simulink model "Simulatore_COMAU_UBHAND_IV_manipolazione_interna"  (Simulink 7.6)
settings: Solver=ode45  StartTime=0.0  StopTime=inf  RelTol=1e-3
values from neighbouring files (symbols this model uses):
  a_f = zeros(4,5)    [KinematicData.m]
  alpha_f = zeros(4,5)    [KinematicData.m]
  d_f = zeros(4,5)    [KinematicData.m]
  dc4_1 = 660    [KinematicData.m]
  T6HAND = [1 0 0 0;0 1 0 0; 0 0 1 357.48+95; 0 0 0 1]    [KinematicData.m]
  teta_d = atan(647.07/130)    [KinematicData.m]
  teta_f = zeros(4,5)    [KinematicData.m]
undefined symbols (not defined in the file or in its folder): a_c, a_w, alpha_c, alpha_w, d_c, d_w, Dt_fingers, teta_c
libraries: simulink x281, dspmtrx3 x171, aerolibutil x163, aerolibconvert2 x64, simulink_extras x46, vrlib x26, aerolibtransform2 x7, dspnetwork x1
(part 14 of 15)

== system Simulatore_COMAU_UBHAND_IV_manipolazione_interna/Work Space Control/Grasp Planner/cilindro/punti di contatto cilindro/object rotation matrix/R_01/rot_y ==
  beta : Inport
  Angle Conversion : aerolibconvert2/Angle Conversion  IU=deg  OU=rad
  Constant{1..3,5} (4 alike) : Constant  Value=0
  Constant4 : Constant
  Create 3x3 Matrix : aerolibutil/Create 3x3 Matrix
  Gain : Gain  Gain=-1
  Trigonometric Function : Trigonometry
  Trigonometric Function1 : Trigonometry  Operator=cos
  Trigonometric Function4 : Trigonometry  Operator=cos
  Trigonometric Function5 : Trigonometry
  roty : Outport
  wires:
  beta/1 -> Angle Conversion/1
  Angle Conversion/1 -> Trigonometric Function1/1, Trigonometric Function/1, Trigonometric Function5/1, Trigonometric Function4/1
  Create 3x3 Matrix/1 -> roty/1
  Constant2/1 -> Create 3x3 Matrix/2
  Trigonometric Function5/1 -> Create 3x3 Matrix/3
  Constant5/1 -> Create 3x3 Matrix/4
  Constant4/1 -> Create 3x3 Matrix/5
  Constant3/1 -> Create 3x3 Matrix/6
  Trigonometric Function/1 -> Gain/1
  Trigonometric Function1/1 -> Create 3x3 Matrix/9
  Trigonometric Function4/1 -> Create 3x3 Matrix/1
  Gain/1 -> Create 3x3 Matrix/7
  Constant1/1 -> Create 3x3 Matrix/8

== system Simulatore_COMAU_UBHAND_IV_manipolazione_interna/Work Space Control/Grasp Planner/cilindro/punti di contatto cilindro/object rotation matrix/R_01/rot_z ==
  gamma : Inport
  Angle Conversion : aerolibconvert2/Angle Conversion  IU=deg  OU=rad
  Constant{,1..3} (4 alike) : Constant  Value=0
  Constant4 : Constant
  Create 3x3 Matrix : aerolibutil/Create 3x3 Matrix
  Gain1 : Gain  Gain=-1
  Trigonometric Function3 : Trigonometry  Operator=cos
  Trigonometric Function4 : Trigonometry  Operator=cos
  Trigonometric Function5 : Trigonometry
  Trigonometric Function6 : Trigonometry
  rotz : Outport
  wires:
  gamma/1 -> Angle Conversion/1
  Angle Conversion/1 -> Trigonometric Function3/1, Trigonometric Function6/1, Trigonometric Function5/1, Trigonometric Function4/1
  Create 3x3 Matrix/1 -> rotz/1
  Trigonometric Function4/1 -> Create 3x3 Matrix/1
  Gain1/1 -> Create 3x3 Matrix/2
  Constant/1 -> Create 3x3 Matrix/3
  Trigonometric Function6/1 -> Create 3x3 Matrix/4
  Trigonometric Function3/1 -> Create 3x3 Matrix/5
  Constant2/1 -> Create 3x3 Matrix/6
  Constant1/1 -> Create 3x3 Matrix/7
  Constant3/1 -> Create 3x3 Matrix/8
  Constant4/1 -> Create 3x3 Matrix/9
  Trigonometric Function5/1 -> Gain1/1

== system Simulatore_COMAU_UBHAND_IV_manipolazione_interna/Work Space Control/Grasp Planner/cilindro/punti di contatto cilindro/object rotation matrix/R_56/rot_x ==
  alpha : Inport
  Angle Conversion : aerolibconvert2/Angle Conversion  IU=deg  OU=rad
  Constant{,1..2,5} (4 alike) : Constant  Value=0
  Constant4 : Constant
  Create 3x3 Matrix : aerolibutil/Create 3x3 Matrix
  Gain : Gain  Gain=-1
  Trigonometric Function : Trigonometry
  Trigonometric Function1 : Trigonometry  Operator=cos
  Trigonometric Function2 : Trigonometry
  Trigonometric Function3 : Trigonometry  Operator=cos
  rotx : Outport
  wires:
  alpha/1 -> Angle Conversion/1
  Angle Conversion/1 -> Trigonometric Function3/1, Trigonometric Function2/1, Trigonometric Function/1, Trigonometric Function1/1
  Create 3x3 Matrix/1 -> rotx/1
  Constant4/1 -> Create 3x3 Matrix/1
  Constant2/1 -> Create 3x3 Matrix/2
  Constant/1 -> Create 3x3 Matrix/3
  Constant5/1 -> Create 3x3 Matrix/4
  Trigonometric Function3/1 -> Create 3x3 Matrix/5
  Trigonometric Function2/1 -> Gain/1
  Gain/1 -> Create 3x3 Matrix/6
  Constant1/1 -> Create 3x3 Matrix/7
  Trigonometric Function/1 -> Create 3x3 Matrix/8
  Trigonometric Function1/1 -> Create 3x3 Matrix/9

== system Simulatore_COMAU_UBHAND_IV_manipolazione_interna/Work Space Control/Grasp Planner/cilindro/punti di contatto cilindro/object rotation matrix/R_56/rot_y ==
  beta : Inport
  Angle Conversion : aerolibconvert2/Angle Conversion  IU=deg  OU=rad
  Constant{1..3,5} (4 alike) : Constant  Value=0
  Constant4 : Constant
  Create 3x3 Matrix : aerolibutil/Create 3x3 Matrix
  Gain : Gain  Gain=-1
  Trigonometric Function : Trigonometry
  Trigonometric Function1 : Trigonometry  Operator=cos
  Trigonometric Function4 : Trigonometry  Operator=cos
  Trigonometric Function5 : Trigonometry
  roty : Outport
  wires:
  Constant1/1 -> Create 3x3 Matrix/8
  Gain/1 -> Create 3x3 Matrix/7
  Trigonometric Function4/1 -> Create 3x3 Matrix/1
  Trigonometric Function1/1 -> Create 3x3 Matrix/9
  Trigonometric Function/1 -> Gain/1
  Constant3/1 -> Create 3x3 Matrix/6
  Constant4/1 -> Create 3x3 Matrix/5
  Constant5/1 -> Create 3x3 Matrix/4
  Trigonometric Function5/1 -> Create 3x3 Matrix/3
  Constant2/1 -> Create 3x3 Matrix/2
  Create 3x3 Matrix/1 -> roty/1
  Angle Conversion/1 -> Trigonometric Function4/1, Trigonometric Function5/1, Trigonometric Function/1, Trigonometric Function1/1
  beta/1 -> Angle Conversion/1

== system Simulatore_COMAU_UBHAND_IV_manipolazione_interna/Work Space Control/Grasp Planner/cilindro/punti di contatto cilindro/object rotation matrix/R_56/rot_z ==
  gamma : Inport
  Angle Conversion : aerolibconvert2/Angle Conversion  IU=deg  OU=rad
  Constant{,1..3} (4 alike) : Constant  Value=0
  Constant4 : Constant
  Create 3x3 Matrix : aerolibutil/Create 3x3 Matrix
  Gain1 : Gain  Gain=-1
  Trigonometric Function3 : Trigonometry  Operator=cos
  Trigonometric Function4 : Trigonometry  Operator=cos
  Trigonometric Function5 : Trigonometry
  Trigonometric Function6 : Trigonometry
  rotz : Outport
  wires:
  Trigonometric Function5/1 -> Gain1/1
  Constant4/1 -> Create 3x3 Matrix/9
  Constant3/1 -> Create 3x3 Matrix/8
  Constant1/1 -> Create 3x3 Matrix/7
  Constant2/1 -> Create 3x3 Matrix/6
  Trigonometric Function3/1 -> Create 3x3 Matrix/5
  Trigonometric Function6/1 -> Create 3x3 Matrix/4
  Constant/1 -> Create 3x3 Matrix/3
  Gain1/1 -> Create 3x3 Matrix/2
  Trigonometric Function4/1 -> Create 3x3 Matrix/1
  Create 3x3 Matrix/1 -> rotz/1
  Angle Conversion/1 -> Trigonometric Function4/1, Trigonometric Function5/1, Trigonometric Function6/1, Trigonometric Function3/1
  gamma/1 -> Angle Conversion/1

== system Simulatore_COMAU_UBHAND_IV_manipolazione_interna/Work Space Control/Grasp Planner/cilindro/punti di contatto cilindro/punti di contatto cilindro/Subsystem1/Subsystem1 ==
  R : Inport
  p : Inport  Port=2
  Constant2 : Constant  Value=[0 0 0 1]  VectorParams1D=off
  Matrix Concatenate2 : Concatenate  Mode=Multidimensional array  ConcatenateDimension=2
  Matrix Concatenate3 : Concatenate  Mode=Multidimensional array
  T : Outport
  wires:
  Constant2/1 -> Matrix Concatenate3/2
  Matrix Concatenate2/1 -> Matrix Concatenate3/1
  R/1 -> Matrix Concatenate2/1
  p/1 -> Matrix Concatenate2/2
  Matrix Concatenate3/1 -> T/1

== system Simulatore_COMAU_UBHAND_IV_manipolazione_interna/Work Space Control/Grasp Planner/cilindro/punti di contatto cilindro/punti di contatto cilindro/Subsystem1/T^-1 ==
  T : Inport
  -R'*v : Gain  Gain=-1
  Constant1 : Constant  Value=[0 0 0 1]  VectorParams1D=off
  Matrix Concatenation : Concatenate  Mode=Multidimensional array  ConcatenateDimension=2
  Matrix Concatenation1 : Concatenate  Mode=Multidimensional array
  R : dspmtrx3/Submatrix  RowSpan=Range of rows  RowStartMode=Index  RowStartIndex=1  RowEndMode=Index  RowEndIndex=3  ColSpan=Range of columns  ColStartMode=Index  ColStartIndex=1  ColEndMode=Index  ColEndIndex=3
  R' : PermuteDimensions
  R'*v : Product  Multiplication=Matrix(*)
  v : dspmtrx3/Submatrix  RowSpan=Range of rows  RowStartMode=Index  RowStartIndex=1  RowEndMode=Index  RowEndIndex=3  ColSpan=One column  ColStartMode=Index  ColStartIndex=4  ColEndMode=Index  ColEndIndex=3
  T^-1 : Outport
  wires:
  T/1 -> R/1, v/1
  R/1 -> R'/1
  R'/1 -> R'*v/1, Matrix Concatenation/1
  v/1 -> R'*v/2
  R'*v/1 -> -R'*v/1
  Constant1/1 -> Matrix Concatenation1/2
  Matrix Concatenation/1 -> Matrix Concatenation1/1
  -R'*v/1 -> Matrix Concatenation/2
  Matrix Concatenation1/1 -> T^-1/1

== system Simulatore_COMAU_UBHAND_IV_manipolazione_interna/Work Space Control/Grasp Planner/cilindro/punti di contatto cilindro/punti di contatto cilindro/Subsystem2/Subsystem1 ==
  R : Inport
  p : Inport  Port=2
  Constant2 : Constant  Value=[0 0 0 1]  VectorParams1D=off
  Matrix Concatenate2 : Concatenate  Mode=Multidimensional array  ConcatenateDimension=2
  Matrix Concatenate3 : Concatenate  Mode=Multidimensional array
  T : Outport
  wires:
  Constant2/1 -> Matrix Concatenate3/2
  Matrix Concatenate2/1 -> Matrix Concatenate3/1
  R/1 -> Matrix Concatenate2/1
  p/1 -> Matrix Concatenate2/2
  Matrix Concatenate3/1 -> T/1

== system Simulatore_COMAU_UBHAND_IV_manipolazione_interna/Work Space Control/Grasp Planner/cilindro/punti di contatto cilindro/punti di contatto cilindro/Subsystem2/T^-1 ==
  T : Inport
  -R'*v : Gain  Gain=-1
  Constant1 : Constant  Value=[0 0 0 1]  VectorParams1D=off
  Matrix Concatenation : Concatenate  Mode=Multidimensional array  ConcatenateDimension=2
  Matrix Concatenation1 : Concatenate  Mode=Multidimensional array
  R : dspmtrx3/Submatrix  RowSpan=Range of rows  RowStartMode=Index  RowStartIndex=1  RowEndMode=Index  RowEndIndex=3  ColSpan=Range of columns  ColStartMode=Index  ColStartIndex=1  ColEndMode=Index  ColEndIndex=3
  R' : PermuteDimensions
  R'*v : Product  Multiplication=Matrix(*)
  v : dspmtrx3/Submatrix  RowSpan=Range of rows  RowStartMode=Index  RowStartIndex=1  RowEndMode=Index  RowEndIndex=3  ColSpan=One column  ColStartMode=Index  ColStartIndex=4  ColEndMode=Index  ColEndIndex=3
  T^-1 : Outport
  wires:
  T/1 -> R/1, v/1
  R/1 -> R'/1
  R'/1 -> R'*v/1, Matrix Concatenation/1
  v/1 -> R'*v/2
  R'*v/1 -> -R'*v/1
  Constant1/1 -> Matrix Concatenation1/2
  Matrix Concatenation/1 -> Matrix Concatenation1/1
  -R'*v/1 -> Matrix Concatenation/2
  Matrix Concatenation1/1 -> T^-1/1

== system Simulatore_COMAU_UBHAND_IV_manipolazione_interna/Work Space Control/Grasp Planner/cilindro/punti di contatto cilindro/punti di contatto cilindro/Subsystem3/Subsystem1 ==
  R : Inport
  p : Inport  Port=2
  Constant2 : Constant  Value=[0 0 0 1]  VectorParams1D=off
  Matrix Concatenate2 : Concatenate  Mode=Multidimensional array  ConcatenateDimension=2
  Matrix Concatenate3 : Concatenate  Mode=Multidimensional array
  T : Outport
  wires:
  Matrix Concatenate3/1 -> T/1
  p/1 -> Matrix Concatenate2/2
  R/1 -> Matrix Concatenate2/1
  Matrix Concatenate2/1 -> Matrix Concatenate3/1
  Constant2/1 -> Matrix Concatenate3/2

== system Simulatore_COMAU_UBHAND_IV_manipolazione_interna/Work Space Control/Grasp Planner/cilindro/punti di contatto cilindro/punti di contatto cilindro/Subsystem3/T^-1 ==
  T : Inport
  -R'*v : Gain  Gain=-1
  Constant1 : Constant  Value=[0 0 0 1]  VectorParams1D=off
  Matrix Concatenation : Concatenate  Mode=Multidimensional array  ConcatenateDimension=2
  Matrix Concatenation1 : Concatenate  Mode=Multidimensional array
  R : dspmtrx3/Submatrix  RowSpan=Range of rows  RowStartMode=Index  RowStartIndex=1  RowEndMode=Index  RowEndIndex=3  ColSpan=Range of columns  ColStartMode=Index  ColStartIndex=1  ColEndMode=Index  ColEndIndex=3
  R' : PermuteDimensions
  R'*v : Product  Multiplication=Matrix(*)
  v : dspmtrx3/Submatrix  RowSpan=Range of rows  RowStartMode=Index  RowStartIndex=1  RowEndMode=Index  RowEndIndex=3  ColSpan=One column  ColStartMode=Index  ColStartIndex=4  ColEndMode=Index  ColEndIndex=3
  T^-1 : Outport
  wires:
  Matrix Concatenation1/1 -> T^-1/1
  -R'*v/1 -> Matrix Concatenation/2
  Matrix Concatenation/1 -> Matrix Concatenation1/1
  Constant1/1 -> Matrix Concatenation1/2
  R'*v/1 -> -R'*v/1
  v/1 -> R'*v/2
  R'/1 -> Matrix Concatenation/1, R'*v/1
  R/1 -> R'/1
  T/1 -> v/1, R/1

== system Simulatore_COMAU_UBHAND_IV_manipolazione_interna/Work Space Control/Grasp Planner/cilindro/punti di contatto cilindro/punti di contatto cilindro/Subsystem4/Subsystem1 ==
  R : Inport
  p : Inport  Port=2
  Constant2 : Constant  Value=[0 0 0 1]  VectorParams1D=off
  Matrix Concatenate2 : Concatenate  Mode=Multidimensional array  ConcatenateDimension=2
  Matrix Concatenate3 : Concatenate  Mode=Multidimensional array
  T : Outport
  wires:
  Constant2/1 -> Matrix Concatenate3/2
  Matrix Concatenate2/1 -> Matrix Concatenate3/1
  R/1 -> Matrix Concatenate2/1
  p/1 -> Matrix Concatenate2/2
  Matrix Concatenate3/1 -> T/1

== system Simulatore_COMAU_UBHAND_IV_manipolazione_interna/Work Space Control/Grasp Planner/cilindro/punti di contatto cilindro/punti di contatto cilindro/Subsystem4/T^-1 ==
  T : Inport
  -R'*v : Gain  Gain=-1
  Constant1 : Constant  Value=[0 0 0 1]  VectorParams1D=off
  Matrix Concatenation : Concatenate  Mode=Multidimensional array  ConcatenateDimension=2
  Matrix Concatenation1 : Concatenate  Mode=Multidimensional array
  R : dspmtrx3/Submatrix  RowSpan=Range of rows  RowStartMode=Index  RowStartIndex=1  RowEndMode=Index  RowEndIndex=3  ColSpan=Range of columns  ColStartMode=Index  ColStartIndex=1  ColEndMode=Index  ColEndIndex=3
  R' : PermuteDimensions
  R'*v : Product  Multiplication=Matrix(*)
  v : dspmtrx3/Submatrix  RowSpan=Range of rows  RowStartMode=Index  RowStartIndex=1  RowEndMode=Index  RowEndIndex=3  ColSpan=One column  ColStartMode=Index  ColStartIndex=4  ColEndMode=Index  ColEndIndex=3
  T^-1 : Outport
  wires:
  T/1 -> R/1, v/1
  R/1 -> R'/1
  R'/1 -> R'*v/1, Matrix Concatenation/1
  v/1 -> R'*v/2
  R'*v/1 -> -R'*v/1
  Constant1/1 -> Matrix Concatenation1/2
  Matrix Concatenation/1 -> Matrix Concatenation1/1
  -R'*v/1 -> Matrix Concatenation/2
  Matrix Concatenation1/1 -> T^-1/1

== system Simulatore_COMAU_UBHAND_IV_manipolazione_interna/Work Space Control/Grasp Planner/cilindro/punti di contatto cilindro/punti di contatto cilindro/Subsystem5/Subsystem1 ==
  R : Inport
  p : Inport  Port=2
  Constant2 : Constant  Value=[0 0 0 1]  VectorParams1D=off
  Matrix Concatenate2 : Concatenate  Mode=Multidimensional array  ConcatenateDimension=2
  Matrix Concatenate3 : Concatenate  Mode=Multidimensional array
  T : Outport
  wires:
  Matrix Concatenate3/1 -> T/1
  p/1 -> Matrix Concatenate2/2
  R/1 -> Matrix Concatenate2/1
  Matrix Concatenate2/1 -> Matrix Concatenate3/1
  Constant2/1 -> Matrix Concatenate3/2

== system Simulatore_COMAU_UBHAND_IV_manipolazione_interna/Work Space Control/Grasp Planner/cilindro/punti di contatto cilindro/punti di contatto cilindro/Subsystem5/T^-1 ==
  T : Inport
  -R'*v : Gain  Gain=-1
  Constant1 : Constant  Value=[0 0 0 1]  VectorParams1D=off
  Matrix Concatenation : Concatenate  Mode=Multidimensional array  ConcatenateDimension=2
  Matrix Concatenation1 : Concatenate  Mode=Multidimensional array
  R : dspmtrx3/Submatrix  RowSpan=Range of rows  RowStartMode=Index  RowStartIndex=1  RowEndMode=Index  RowEndIndex=3  ColSpan=Range of columns  ColStartMode=Index  ColStartIndex=1  ColEndMode=Index  ColEndIndex=3
  R' : PermuteDimensions
  R'*v : Product  Multiplication=Matrix(*)
  v : dspmtrx3/Submatrix  RowSpan=Range of rows  RowStartMode=Index  RowStartIndex=1  RowEndMode=Index  RowEndIndex=3  ColSpan=One column  ColStartMode=Index  ColStartIndex=4  ColEndMode=Index  ColEndIndex=3
  T^-1 : Outport
  wires:
  Matrix Concatenation1/1 -> T^-1/1
  -R'*v/1 -> Matrix Concatenation/2
  Matrix Concatenation/1 -> Matrix Concatenation1/1
  Constant1/1 -> Matrix Concatenation1/2
  R'*v/1 -> -R'*v/1
  v/1 -> R'*v/2
  R'/1 -> Matrix Concatenation/1, R'*v/1
  R/1 -> R'/1
  T/1 -> v/1, R/1

== system Simulatore_COMAU_UBHAND_IV_manipolazione_interna/Work Space Control/Grasp Planner/sfera/punti di contatto sfera/object rotation matrix/R/rot_x ==
  alpha : Inport
  Angle Conversion : aerolibconvert2/Angle Conversion  IU=deg  OU=rad
  Constant{,1..2,5} (4 alike) : Constant  Value=0
  Constant4 : Constant
  Create 3x3 Matrix : aerolibutil/Create 3x3 Matrix
  Gain : Gain  Gain=-1
  Trigonometric Function : Trigonometry
  Trigonometric Function1 : Trigonometry  Operator=cos
  Trigonometric Function2 : Trigonometry
  Trigonometric Function3 : Trigonometry  Operator=cos
  rotx : Outport
  wires:
  Trigonometric Function1/1 -> Create 3x3 Matrix/9
  Trigonometric Function/1 -> Create 3x3 Matrix/8
  Constant1/1 -> Create 3x3 Matrix/7
  Gain/1 -> Create 3x3 Matrix/6
  Trigonometric Function2/1 -> Gain/1
  Trigonometric Function3/1 -> Create 3x3 Matrix/5
  Constant5/1 -> Create 3x3 Matrix/4
  Constant/1 -> Create 3x3 Matrix/3
  Constant2/1 -> Create 3x3 Matrix/2
  Constant4/1 -> Create 3x3 Matrix/1
  Create 3x3 Matrix/1 -> rotx/1
  Angle Conversion/1 -> Trigonometric Function1/1, Trigonometric Function/1, Trigonometric Function2/1, Trigonometric Function3/1
  alpha/1 -> Angle Conversion/1

== system Simulatore_COMAU_UBHAND_IV_manipolazione_interna/Work Space Control/Grasp Planner/sfera/punti di contatto sfera/object rotation matrix/R/rot_y ==
  beta : Inport
  Angle Conversion : aerolibconvert2/Angle Conversion  IU=deg  OU=rad
  Constant{1..3,5} (4 alike) : Constant  Value=0
  Constant4 : Constant
  Create 3x3 Matrix : aerolibutil/Create 3x3 Matrix
  Gain : Gain  Gain=-1
  Trigonometric Function : Trigonometry
  Trigonometric Function1 : Trigonometry  Operator=cos
  Trigonometric Function4 : Trigonometry  Operator=cos
  Trigonometric Function5 : Trigonometry
  roty : Outport
  wires:
  beta/1 -> Angle Conversion/1
  Angle Conversion/1 -> Trigonometric Function1/1, Trigonometric Function/1, Trigonometric Function5/1, Trigonometric Function4/1
  Create 3x3 Matrix/1 -> roty/1
  Constant2/1 -> Create 3x3 Matrix/2
  Trigonometric Function5/1 -> Create 3x3 Matrix/3
  Constant5/1 -> Create 3x3 Matrix/4
  Constant4/1 -> Create 3x3 Matrix/5
  Constant3/1 -> Create 3x3 Matrix/6
  Trigonometric Function/1 -> Gain/1
  Trigonometric Function1/1 -> Create 3x3 Matrix/9
  Trigonometric Function4/1 -> Create 3x3 Matrix/1
  Gain/1 -> Create 3x3 Matrix/7
  Constant1/1 -> Create 3x3 Matrix/8

== system Simulatore_COMAU_UBHAND_IV_manipolazione_interna/Work Space Control/Grasp Planner/sfera/punti di contatto sfera/object rotation matrix/R/rot_z ==
  gamma : Inport
  Angle Conversion : aerolibconvert2/Angle Conversion  IU=deg  OU=rad
  Constant{,1..3} (4 alike) : Constant  Value=0
  Constant4 : Constant
  Create 3x3 Matrix : aerolibutil/Create 3x3 Matrix
  Gain1 : Gain  Gain=-1
  Trigonometric Function3 : Trigonometry  Operator=cos
  Trigonometric Function4 : Trigonometry  Operator=cos
  Trigonometric Function5 : Trigonometry
  Trigonometric Function6 : Trigonometry
  rotz : Outport
  wires:
  gamma/1 -> Angle Conversion/1
  Angle Conversion/1 -> Trigonometric Function3/1, Trigonometric Function6/1, Trigonometric Function5/1, Trigonometric Function4/1
  Create 3x3 Matrix/1 -> rotz/1
  Trigonometric Function4/1 -> Create 3x3 Matrix/1
  Gain1/1 -> Create 3x3 Matrix/2
  Constant/1 -> Create 3x3 Matrix/3
  Trigonometric Function6/1 -> Create 3x3 Matrix/4
  Trigonometric Function3/1 -> Create 3x3 Matrix/5
  Constant2/1 -> Create 3x3 Matrix/6
  Constant1/1 -> Create 3x3 Matrix/7
  Constant3/1 -> Create 3x3 Matrix/8
  Constant4/1 -> Create 3x3 Matrix/9
  Trigonometric Function5/1 -> Gain1/1

== system Simulatore_COMAU_UBHAND_IV_manipolazione_interna/Work Space Control/Grasp Planner/sfera/punti di contatto sfera/object rotation matrix/R_01/rot_x ==
  alpha : Inport
  Angle Conversion : aerolibconvert2/Angle Conversion  IU=deg  OU=rad
  Constant{,1..2,5} (4 alike) : Constant  Value=0
  Constant4 : Constant
  Create 3x3 Matrix : aerolibutil/Create 3x3 Matrix
  Gain : Gain  Gain=-1
  Trigonometric Function : Trigonometry
  Trigonometric Function1 : Trigonometry  Operator=cos
  Trigonometric Function2 : Trigonometry
  Trigonometric Function3 : Trigonometry  Operator=cos
  rotx : Outport
  wires:
  Trigonometric Function1/1 -> Create 3x3 Matrix/9
  Trigonometric Function/1 -> Create 3x3 Matrix/8
  Constant1/1 -> Create 3x3 Matrix/7
  Gain/1 -> Create 3x3 Matrix/6
  Trigonometric Function2/1 -> Gain/1
  Trigonometric Function3/1 -> Create 3x3 Matrix/5
  Constant5/1 -> Create 3x3 Matrix/4
  Constant/1 -> Create 3x3 Matrix/3
  Constant2/1 -> Create 3x3 Matrix/2
  Constant4/1 -> Create 3x3 Matrix/1
  Create 3x3 Matrix/1 -> rotx/1
  Angle Conversion/1 -> Trigonometric Function1/1, Trigonometric Function/1, Trigonometric Function2/1, Trigonometric Function3/1
  alpha/1 -> Angle Conversion/1

== system Simulatore_COMAU_UBHAND_IV_manipolazione_interna/Work Space Control/Grasp Planner/sfera/punti di contatto sfera/object rotation matrix/R_01/rot_y ==
  beta : Inport
  Angle Conversion : aerolibconvert2/Angle Conversion  IU=deg  OU=rad
  Constant{1..3,5} (4 alike) : Constant  Value=0
  Constant4 : Constant
  Create 3x3 Matrix : aerolibutil/Create 3x3 Matrix
  Gain : Gain  Gain=-1
  Trigonometric Function : Trigonometry
  Trigonometric Function1 : Trigonometry  Operator=cos
  Trigonometric Function4 : Trigonometry  Operator=cos
  Trigonometric Function5 : Trigonometry
  roty : Outport
  wires:
  beta/1 -> Angle Conversion/1
  Angle Conversion/1 -> Trigonometric Function1/1, Trigonometric Function/1, Trigonometric Function5/1, Trigonometric Function4/1
  Create 3x3 Matrix/1 -> roty/1
  Constant2/1 -> Create 3x3 Matrix/2
  Trigonometric Function5/1 -> Create 3x3 Matrix/3
  Constant5/1 -> Create 3x3 Matrix/4
  Constant4/1 -> Create 3x3 Matrix/5
  Constant3/1 -> Create 3x3 Matrix/6
  Trigonometric Function/1 -> Gain/1
  Trigonometric Function1/1 -> Create 3x3 Matrix/9
  Trigonometric Function4/1 -> Create 3x3 Matrix/1
  Gain/1 -> Create 3x3 Matrix/7
  Constant1/1 -> Create 3x3 Matrix/8

== system Simulatore_COMAU_UBHAND_IV_manipolazione_interna/Work Space Control/Grasp Planner/sfera/punti di contatto sfera/object rotation matrix/R_01/rot_z ==
  gamma : Inport
  Angle Conversion : aerolibconvert2/Angle Conversion  IU=deg  OU=rad
  Constant{,1..3} (4 alike) : Constant  Value=0
  Constant4 : Constant
  Create 3x3 Matrix : aerolibutil/Create 3x3 Matrix
  Gain1 : Gain  Gain=-1
  Trigonometric Function3 : Trigonometry  Operator=cos
  Trigonometric Function4 : Trigonometry  Operator=cos
  Trigonometric Function5 : Trigonometry
  Trigonometric Function6 : Trigonometry
  rotz : Outport
  wires:
  gamma/1 -> Angle Conversion/1
  Angle Conversion/1 -> Trigonometric Function3/1, Trigonometric Function6/1, Trigonometric Function5/1, Trigonometric Function4/1
  Create 3x3 Matrix/1 -> rotz/1
  Trigonometric Function4/1 -> Create 3x3 Matrix/1
  Gain1/1 -> Create 3x3 Matrix/2
  Constant/1 -> Create 3x3 Matrix/3
  Trigonometric Function6/1 -> Create 3x3 Matrix/4
  Trigonometric Function3/1 -> Create 3x3 Matrix/5
  Constant2/1 -> Create 3x3 Matrix/6
  Constant1/1 -> Create 3x3 Matrix/7
  Constant3/1 -> Create 3x3 Matrix/8
  Constant4/1 -> Create 3x3 Matrix/9
  Trigonometric Function5/1 -> Gain1/1

== system Simulatore_COMAU_UBHAND_IV_manipolazione_interna/Work Space Control/Grasp Planner/sfera/punti di contatto sfera/object rotation matrix/R_56/rot_x ==
  alpha : Inport
  Angle Conversion : aerolibconvert2/Angle Conversion  IU=deg  OU=rad
  Constant{,1..2,5} (4 alike) : Constant  Value=0
  Constant4 : Constant
  Create 3x3 Matrix : aerolibutil/Create 3x3 Matrix
  Gain : Gain  Gain=-1
  Trigonometric Function : Trigonometry
  Trigonometric Function1 : Trigonometry  Operator=cos
  Trigonometric Function2 : Trigonometry
  Trigonometric Function3 : Trigonometry  Operator=cos
  rotx : Outport
  wires:
  alpha/1 -> Angle Conversion/1
  Angle Conversion/1 -> Trigonometric Function3/1, Trigonometric Function2/1, Trigonometric Function/1, Trigonometric Function1/1
  Create 3x3 Matrix/1 -> rotx/1
  Constant4/1 -> Create 3x3 Matrix/1
  Constant2/1 -> Create 3x3 Matrix/2
  Constant/1 -> Create 3x3 Matrix/3
  Constant5/1 -> Create 3x3 Matrix/4
  Trigonometric Function3/1 -> Create 3x3 Matrix/5
  Trigonometric Function2/1 -> Gain/1
  Gain/1 -> Create 3x3 Matrix/6
  Constant1/1 -> Create 3x3 Matrix/7
  Trigonometric Function/1 -> Create 3x3 Matrix/8
  Trigonometric Function1/1 -> Create 3x3 Matrix/9

== system Simulatore_COMAU_UBHAND_IV_manipolazione_interna/Work Space Control/Grasp Planner/sfera/punti di contatto sfera/object rotation matrix/R_56/rot_y ==
  beta : Inport
  Angle Conversion : aerolibconvert2/Angle Conversion  IU=deg  OU=rad
  Constant{1..3,5} (4 alike) : Constant  Value=0
  Constant4 : Constant
  Create 3x3 Matrix : aerolibutil/Create 3x3 Matrix
  Gain : Gain  Gain=-1
  Trigonometric Function : Trigonometry
  Trigonometric Function1 : Trigonometry  Operator=cos
  Trigonometric Function4 : Trigonometry  Operator=cos
  Trigonometric Function5 : Trigonometry
  roty : Outport
  wires:
  Constant1/1 -> Create 3x3 Matrix/8
  Gain/1 -> Create 3x3 Matrix/7
  Trigonometric Function4/1 -> Create 3x3 Matrix/1
  Trigonometric Function1/1 -> Create 3x3 Matrix/9
  Trigonometric Function/1 -> Gain/1
  Constant3/1 -> Create 3x3 Matrix/6
  Constant4/1 -> Create 3x3 Matrix/5
  Constant5/1 -> Create 3x3 Matrix/4
  Trigonometric Function5/1 -> Create 3x3 Matrix/3
  Constant2/1 -> Create 3x3 Matrix/2
  Create 3x3 Matrix/1 -> roty/1
  Angle Conversion/1 -> Trigonometric Function4/1, Trigonometric Function5/1, Trigonometric Function/1, Trigonometric Function1/1
  beta/1 -> Angle Conversion/1

== system Simulatore_COMAU_UBHAND_IV_manipolazione_interna/Work Space Control/Grasp Planner/sfera/punti di contatto sfera/object rotation matrix/R_56/rot_z ==
  gamma : Inport
  Angle Conversion : aerolibconvert2/Angle Conversion  IU=deg  OU=rad
  Constant{,1..3} (4 alike) : Constant  Value=0
  Constant4 : Constant
  Create 3x3 Matrix : aerolibutil/Create 3x3 Matrix
  Gain1 : Gain  Gain=-1
  Trigonometric Function3 : Trigonometry  Operator=cos
  Trigonometric Function4 : Trigonometry  Operator=cos
  Trigonometric Function5 : Trigonometry
  Trigonometric Function6 : Trigonometry
  rotz : Outport
  wires:
  Trigonometric Function5/1 -> Gain1/1
  Constant4/1 -> Create 3x3 Matrix/9
  Constant3/1 -> Create 3x3 Matrix/8
  Constant1/1 -> Create 3x3 Matrix/7
  Constant2/1 -> Create 3x3 Matrix/6
  Trigonometric Function3/1 -> Create 3x3 Matrix/5
  Trigonometric Function6/1 -> Create 3x3 Matrix/4
  Constant/1 -> Create 3x3 Matrix/3
  Gain1/1 -> Create 3x3 Matrix/2
  Trigonometric Function4/1 -> Create 3x3 Matrix/1
  Create 3x3 Matrix/1 -> rotz/1
  Angle Conversion/1 -> Trigonometric Function4/1, Trigonometric Function5/1, Trigonometric Function6/1, Trigonometric Function3/1
  gamma/1 -> Angle Conversion/1

== system Simulatore_COMAU_UBHAND_IV_manipolazione_interna/Work Space Control/Grasp Planner/sfera/punti di contatto sfera/punti di contatto sfera/R/rot_x ==
  alpha : Inport
  Angle Conversion : aerolibconvert2/Angle Conversion  IU=deg  OU=rad
  Constant{,1..2,5} (4 alike) : Constant  Value=0
  Constant4 : Constant
  Create 3x3 Matrix : aerolibutil/Create 3x3 Matrix
  Gain : Gain  Gain=-1
  Trigonometric Function : Trigonometry
  Trigonometric Function1 : Trigonometry  Operator=cos
  Trigonometric Function2 : Trigonometry
  Trigonometric Function3 : Trigonometry  Operator=cos
  rotx : Outport
  wires:
  alpha/1 -> Angle Conversion/1
  Angle Conversion/1 -> Trigonometric Function3/1, Trigonometric Function2/1, Trigonometric Function/1, Trigonometric Function1/1
  Create 3x3 Matrix/1 -> rotx/1
  Constant4/1 -> Create 3x3 Matrix/1
  Constant2/1 -> Create 3x3 Matrix/2
  Constant/1 -> Create 3x3 Matrix/3
  Constant5/1 -> Create 3x3 Matrix/4
  Trigonometric Function3/1 -> Create 3x3 Matrix/5
  Trigonometric Function2/1 -> Gain/1
  Gain/1 -> Create 3x3 Matrix/6
  Constant1/1 -> Create 3x3 Matrix/7
  Trigonometric Function/1 -> Create 3x3 Matrix/8
  Trigonometric Function1/1 -> Create 3x3 Matrix/9

== system Simulatore_COMAU_UBHAND_IV_manipolazione_interna/Work Space Control/Grasp Planner/sfera/punti di contatto sfera/punti di contatto sfera/R/rot_y ==
  beta : Inport
  Angle Conversion : aerolibconvert2/Angle Conversion  IU=deg  OU=rad
  Constant{1..3,5} (4 alike) : Constant  Value=0
  Constant4 : Constant
  Create 3x3 Matrix : aerolibutil/Create 3x3 Matrix
  Gain : Gain  Gain=-1
  Trigonometric Function : Trigonometry
  Trigonometric Function1 : Trigonometry  Operator=cos
  Trigonometric Function4 : Trigonometry  Operator=cos
  Trigonometric Function5 : Trigonometry
  roty : Outport
  wires:
  Constant1/1 -> Create 3x3 Matrix/8
  Gain/1 -> Create 3x3 Matrix/7
  Trigonometric Function4/1 -> Create 3x3 Matrix/1
  Trigonometric Function1/1 -> Create 3x3 Matrix/9
  Trigonometric Function/1 -> Gain/1
  Constant3/1 -> Create 3x3 Matrix/6
  Constant4/1 -> Create 3x3 Matrix/5
  Constant5/1 -> Create 3x3 Matrix/4
  Trigonometric Function5/1 -> Create 3x3 Matrix/3
  Constant2/1 -> Create 3x3 Matrix/2
  Create 3x3 Matrix/1 -> roty/1
  Angle Conversion/1 -> Trigonometric Function4/1, Trigonometric Function5/1, Trigonometric Function/1, Trigonometric Function1/1
  beta/1 -> Angle Conversion/1

== system Simulatore_COMAU_UBHAND_IV_manipolazione_interna/Work Space Control/Grasp Planner/sfera/punti di contatto sfera/punti di contatto sfera/R/rot_z ==
  gamma : Inport
  Angle Conversion : aerolibconvert2/Angle Conversion  IU=deg  OU=rad
  Constant{,1..3} (4 alike) : Constant  Value=0
  Constant4 : Constant
  Create 3x3 Matrix : aerolibutil/Create 3x3 Matrix
  Gain1 : Gain  Gain=-1
  Trigonometric Function3 : Trigonometry  Operator=cos
  Trigonometric Function4 : Trigonometry  Operator=cos
  Trigonometric Function5 : Trigonometry
  Trigonometric Function6 : Trigonometry
  rotz : Outport
  wires:
  Trigonometric Function5/1 -> Gain1/1
  Constant4/1 -> Create 3x3 Matrix/9
  Constant3/1 -> Create 3x3 Matrix/8
  Constant1/1 -> Create 3x3 Matrix/7
  Constant2/1 -> Create 3x3 Matrix/6
  Trigonometric Function3/1 -> Create 3x3 Matrix/5
  Trigonometric Function6/1 -> Create 3x3 Matrix/4
  Constant/1 -> Create 3x3 Matrix/3
  Gain1/1 -> Create 3x3 Matrix/2
  Trigonometric Function4/1 -> Create 3x3 Matrix/1
  Create 3x3 Matrix/1 -> rotz/1
  Angle Conversion/1 -> Trigonometric Function4/1, Trigonometric Function5/1, Trigonometric Function6/1, Trigonometric Function3/1
  gamma/1 -> Angle Conversion/1

== system Simulatore_COMAU_UBHAND_IV_manipolazione_interna/Work Space Control/Inverse_kinematic/Inverse_kinematic_comau/R_0index/T_0index/Direct_kinematic_index/Subsystem ==
  q : Inport
  alpha : Inport  Port=2
  a : Inport  Port=3
  A_01 : aerolibutil/Create 3x3 Matrix
  Constant : Constant  Value=0
  Constant1 : Constant  Value=[0 0 0 1]  VectorParams1D=off
  Constant5 : Constant  Value=0
  Display : Display
  Gain : Gain  Gain=-1
  Gain1 : Gain  Gain=-1
  Matrix Concatenation : Concatenate  Mode=Multidimensional array  ConcatenateDimension=2
  Matrix Concatenation1 : Concatenate  Mode=Multidimensional array
  Product{,1..5} (6 alike) : Product  RndMeth=Zero
  Trigonometric Function{,1,6..8,11..12} (7 alike) : Trigonometry  Operator=cos
  Trigonometric Function{2..5,9..10,13} (7 alike) : Trigonometry
  Vector Concatenate : Concatenate  NumInputs=3  Mode=Multidimensional array
  Out1 : Outport
  wires:
  Matrix Concatenation1/1 -> Display/1, Out1/1
  Constant1/1 -> Matrix Concatenation1/2
  Matrix Concatenation/1 -> Matrix Concatenation1/1
  Vector Concatenate/1 -> Matrix Concatenation/2
  A_01/1 -> Matrix Concatenation/1
  Constant5/1 -> Vector Concatenate/3
  Product5/1 -> Vector Concatenate/2
  Product4/1 -> Vector Concatenate/1
  Trigonometric Function13/1 -> Product5/2
  Trigonometric Function12/1 -> Product4/2
  a/1 -> Product5/1, Product4/1
  Trigonometric Function11/1 -> A_01/9
  Trigonometric Function10/1 -> A_01/8
  Constant/1 -> A_01/7
  Gain1/1 -> A_01/6
  Product2/1 -> A_01/5
  Trigonometric Function5/1 -> A_01/4
  Product1/1 -> A_01/3
  Gain/1 -> A_01/2
  Trigonometric Function/1 -> A_01/1
  Product/1 -> Gain/1
  Product3/1 -> Gain1/1
  Trigonometric Function9/1 -> Product3/2
  Trigonometric Function8/1 -> Product3/1
  Trigonometric Function7/1 -> Product2/2
  Trigonometric Function6/1 -> Product2/1
  Trigonometric Function4/1 -> Product1/2
  Trigonometric Function3/1 -> Product1/1
  alpha/1 -> Trigonometric Function11/1, Trigonometric Function10/1, Trigonometric Function9/1, Trigonometric Function7/1, Trigonometric Function4/1, Trigonometric Function1/1
  q/1 -> Trigonometric Function13/1, Trigonometric Function12/1, Trigonometric Function8/1, Trigonometric Function6/1, Trigonometric Function5/1, Trigonometric Function3/1, Trigonometric Function2/1, Trigonometric Function/1
  Trigonometric Function1/1 -> Product/2
  Trigonometric Function2/1 -> Product/1

== system Simulatore_COMAU_UBHAND_IV_manipolazione_interna/Work Space Control/Inverse_kinematic/Inverse_kinematic_comau/R_0index/T_0index/Direct_kinematic_index/Subsystem1 ==
  q : Inport
  alpha : Inport  Port=2
  a : Inport  Port=3
  A_01 : aerolibutil/Create 3x3 Matrix
  Constant : Constant  Value=0
  Constant1 : Constant  Value=[0 0 0 1]  VectorParams1D=off
  Constant5 : Constant  Value=0
  Display : Display
  Gain : Gain  Gain=-1
  Gain1 : Gain  Gain=-1
  Matrix Concatenation : Concatenate  Mode=Multidimensional array  ConcatenateDimension=2
  Matrix Concatenation1 : Concatenate  Mode=Multidimensional array
  Product{,1..5} (6 alike) : Product  RndMeth=Zero
  Trigonometric Function{,1,6..8,11..12} (7 alike) : Trigonometry  Operator=cos
  Trigonometric Function{2..5,9..10,13} (7 alike) : Trigonometry
  Vector Concatenate : Concatenate  NumInputs=3  Mode=Multidimensional array
  Out1 : Outport
  wires:
  Trigonometric Function2/1 -> Product/1
  Trigonometric Function1/1 -> Product/2
  q/1 -> Trigonometric Function/1, Trigonometric Function2/1, Trigonometric Function3/1, Trigonometric Function5/1, Trigonometric Function6/1, Trigonometric Function8/1, Trigonometric Function12/1, Trigonometric Function13/1
  alpha/1 -> Trigonometric Function1/1, Trigonometric Function4/1, Trigonometric Function7/1, Trigonometric Function9/1, Trigonometric Function10/1, Trigonometric Function11/1
  Trigonometric Function3/1 -> Product1/1
  Trigonometric Function4/1 -> Product1/2
  Trigonometric Function6/1 -> Product2/1
  Trigonometric Function7/1 -> Product2/2
  Trigonometric Function8/1 -> Product3/1
  Trigonometric Function9/1 -> Product3/2
  Product3/1 -> Gain1/1
  Product/1 -> Gain/1
  Trigonometric Function/1 -> A_01/1
  Gain/1 -> A_01/2
  Product1/1 -> A_01/3
  Trigonometric Function5/1 -> A_01/4
  Product2/1 -> A_01/5
  Gain1/1 -> A_01/6
  Constant/1 -> A_01/7
  Trigonometric Function10/1 -> A_01/8
  Trigonometric Function11/1 -> A_01/9
  a/1 -> Product5/1, Product4/1
  Trigonometric Function12/1 -> Product4/2
  Trigonometric Function13/1 -> Product5/2
  Product4/1 -> Vector Concatenate/1
  Product5/1 -> Vector Concatenate/2
  Constant5/1 -> Vector Concatenate/3
  A_01/1 -> Matrix Concatenation/1
  Vector Concatenate/1 -> Matrix Concatenation/2
  Matrix Concatenation/1 -> Matrix Concatenation1/1
  Constant1/1 -> Matrix Concatenation1/2
  Matrix Concatenation1/1 -> Out1/1, Display/1

== system Simulatore_COMAU_UBHAND_IV_manipolazione_interna/Work Space Control/Inverse_kinematic/Inverse_kinematic_comau/R_0index/T_0index/Direct_kinematic_index/Subsystem2 ==
  q : Inport
  alpha : Inport  Port=2
  a : Inport  Port=3
  A_01 : aerolibutil/Create 3x3 Matrix
  Constant : Constant  Value=0
  Constant1 : Constant  Value=[0 0 0 1]  VectorParams1D=off
  Constant5 : Constant  Value=0
  Display : Display
  Gain : Gain  Gain=-1
  Gain1 : Gain  Gain=-1
  Matrix Concatenation : Concatenate  Mode=Multidimensional array  ConcatenateDimension=2
  Matrix Concatenation1 : Concatenate  Mode=Multidimensional array
  Product{,1..5} (6 alike) : Product  RndMeth=Zero
  Trigonometric Function{,1,6..8,11..12} (7 alike) : Trigonometry  Operator=cos
  Trigonometric Function{2..5,9..10,13} (7 alike) : Trigonometry
  Vector Concatenate : Concatenate  NumInputs=3  Mode=Multidimensional array
  Out1 : Outport
  wires:
  Matrix Concatenation1/1 -> Display/1, Out1/1
  Constant1/1 -> Matrix Concatenation1/2
  Matrix Concatenation/1 -> Matrix Concatenation1/1
  Vector Concatenate/1 -> Matrix Concatenation/2
  A_01/1 -> Matrix Concatenation/1
  Constant5/1 -> Vector Concatenate/3
  Product5/1 -> Vector Concatenate/2
  Product4/1 -> Vector Concatenate/1
  Trigonometric Function13/1 -> Product5/2
  Trigonometric Function12/1 -> Product4/2
  a/1 -> Product5/1, Product4/1
  Trigonometric Function11/1 -> A_01/9
  Trigonometric Function10/1 -> A_01/8
  Constant/1 -> A_01/7
  Gain1/1 -> A_01/6
  Product2/1 -> A_01/5
  Trigonometric Function5/1 -> A_01/4
  Product1/1 -> A_01/3
  Gain/1 -> A_01/2
  Trigonometric Function/1 -> A_01/1
  Product/1 -> Gain/1
  Product3/1 -> Gain1/1
  Trigonometric Function9/1 -> Product3/2
  Trigonometric Function8/1 -> Product3/1
  Trigonometric Function7/1 -> Product2/2
  Trigonometric Function6/1 -> Product2/1
  Trigonometric Function4/1 -> Product1/2
  Trigonometric Function3/1 -> Product1/1
  alpha/1 -> Trigonometric Function11/1, Trigonometric Function10/1, Trigonometric Function9/1, Trigonometric Function7/1, Trigonometric Function4/1, Trigonometric Function1/1
  q/1 -> Trigonometric Function13/1, Trigonometric Function12/1, Trigonometric Function8/1, Trigonometric Function6/1, Trigonometric Function5/1, Trigonometric Function3/1, Trigonometric Function2/1, Trigonometric Function/1
  Trigonometric Function1/1 -> Product/2
  Trigonometric Function2/1 -> Product/1

== system Simulatore_COMAU_UBHAND_IV_manipolazione_interna/Work Space Control/Inverse_kinematic/Inverse_kinematic_comau/R_0index/T_0index/Direct_kinematic_index/Subsystem3 ==
  q : Inport
  alpha : Inport  Port=2
  a : Inport  Port=3
  A_01 : aerolibutil/Create 3x3 Matrix
  Constant : Constant  Value=0
  Constant1 : Constant  Value=[0 0 0 1]  VectorParams1D=off
  Constant5 : Constant  Value=0
  Display : Display
  Gain : Gain  Gain=-1
  Gain1 : Gain  Gain=-1
  Matrix Concatenation : Concatenate  Mode=Multidimensional array  ConcatenateDimension=2
  Matrix Concatenation1 : Concatenate  Mode=Multidimensional array
  Product{,1..5} (6 alike) : Product  RndMeth=Zero
  Trigonometric Function{,1,6..8,11..12} (7 alike) : Trigonometry  Operator=cos
  Trigonometric Function{2..5,9..10,13} (7 alike) : Trigonometry
  Vector Concatenate : Concatenate  NumInputs=3  Mode=Multidimensional array
  Out1 : Outport
  wires:
  Trigonometric Function2/1 -> Product/1
  Trigonometric Function1/1 -> Product/2
  q/1 -> Trigonometric Function/1, Trigonometric Function2/1, Trigonometric Function3/1, Trigonometric Function5/1, Trigonometric Function6/1, Trigonometric Function8/1, Trigonometric Function12/1, Trigonometric Function13/1
  alpha/1 -> Trigonometric Function1/1, Trigonometric Function4/1, Trigonometric Function7/1, Trigonometric Function9/1, Trigonometric Function10/1, Trigonometric Function11/1
  Trigonometric Function3/1 -> Product1/1
  Trigonometric Function4/1 -> Product1/2
  Trigonometric Function6/1 -> Product2/1
  Trigonometric Function7/1 -> Product2/2
  Trigonometric Function8/1 -> Product3/1
  Trigonometric Function9/1 -> Product3/2
  Product3/1 -> Gain1/1
  Product/1 -> Gain/1
  Trigonometric Function/1 -> A_01/1
  Gain/1 -> A_01/2
  Product1/1 -> A_01/3
  Trigonometric Function5/1 -> A_01/4
  Product2/1 -> A_01/5
  Gain1/1 -> A_01/6
  Constant/1 -> A_01/7
  Trigonometric Function10/1 -> A_01/8
  Trigonometric Function11/1 -> A_01/9
  a/1 -> Product5/1, Product4/1
  Trigonometric Function12/1 -> Product4/2
  Trigonometric Function13/1 -> Product5/2
  Product4/1 -> Vector Concatenate/1
  Product5/1 -> Vector Concatenate/2
  Constant5/1 -> Vector Concatenate/3
  A_01/1 -> Matrix Concatenation/1
  Vector Concatenate/1 -> Matrix Concatenation/2
  Matrix Concatenation/1 -> Matrix Concatenation1/1
  Constant1/1 -> Matrix Concatenation1/2
  Matrix Concatenation1/1 -> Out1/1, Display/1

== system Simulatore_COMAU_UBHAND_IV_manipolazione_interna/Work Space Control/Inverse_kinematic/Inverse_kinematic_comau/R_0index/T_0index/Direct_kinematic_wrist/T_6_8 ==
  wrist_angles[1..2] : Inport
  R_0H : Inport  Port=2
  Add : Sum
  Add1 : Sum
  Constant : Constant  Value=[alpha_w(1) alpha_w(2)]
  Constant1 : Constant  Value=0
  Constant2 : Constant  Value=[a_w(1) a_w(2)]
  Constant3 : Constant  Value=0
  Degrees to Radians : simulink_extras/Transformations/Degrees to Radians
  Demux : Demux  Outputs=2
  Demux1 : Demux  Outputs=2
  Demux2 : Demux  Outputs=2
  Display3 : Display
  Matrix Multiply : Product  Multiplication=Matrix(*)
  Matrix Multiply1 : Product  Multiplication=Matrix(*)
  Matrix Multiply2 : Product  Multiplication=Matrix(*)
  R_0EE : dspmtrx3/Submatrix  RowSpan=Range of rows  RowStartMode=Index  RowStartIndex=1  RowEndMode=Index  RowEndIndex=3  ColSpan=Range of columns  ColStartMode=Index  ColStartIndex=1  ColEndMode=Index  ColEndIndex=3
  R_0EE1 : dspmtrx3/Submatrix  RowSpan=Range of rows  RowStartMode=Index  RowStartIndex=1  RowEndMode=Index  RowEndIndex=3  ColSpan=Range of columns  ColStartMode=Index  ColStartIndex=1  ColEndMode=Index  ColEndIndex=3
  Subsystem : Subsystem
  Subsystem1 : Subsystem
  wrist_reference_ : Subsystem
  R_08 : Outport
  wires:
  wrist_angles[1..2]/1 -> Display3/1, Degrees to Radians/1
  Degrees to Radians/1 -> Demux/1
  Demux1/2 -> Subsystem1/2(alpha)
  Demux1/1 -> Subsystem/2(alpha)
  Demux2/2 -> Subsystem1/3(a)
  Demux2/1 -> Subsystem/3(a)
  Constant2/1 -> Demux2/1
  Matrix Multiply/1 -> R_08/1
  Subsystem1/1(Out1) -> R_0EE1/1
  Constant/1 -> Demux1/1
  Subsystem/1(Out1) -> R_0EE/1
  Matrix Multiply1/1 -> Matrix Multiply/1
  Constant1/1 -> Add/2
  Demux/1 -> Add/1
  Add/1 -> Subsystem/1(q)
  Constant3/1 -> Add1/2
  Demux/2 -> Add1/1
  Add1/1 -> Subsystem1/1(q)
  wrist_reference_/1(R_HW) -> Matrix Multiply2/2
  Matrix Multiply2/1 -> Matrix Multiply1/1
  R_0H/1 -> Matrix Multiply2/1
  R_0EE/1 -> Matrix Multiply1/2
  R_0EE1/1 -> Matrix Multiply/2

== system Simulatore_COMAU_UBHAND_IV_manipolazione_interna/Work Space Control/Inverse_kinematic/Inverse_kinematic_comau/R_0index/T_6_index_^-1/Direct_kinematic_index1/Subsystem ==
  q : Inport
  alpha : Inport  Port=2
  a : Inport  Port=3
  A_01 : aerolibutil/Create 3x3 Matrix
  Constant : Constant  Value=0
  Constant1 : Constant  Value=[0 0 0 1]  VectorParams1D=off
  Constant5 : Constant  Value=0
  Display : Display
  Gain : Gain  Gain=-1
  Gain1 : Gain  Gain=-1
  Matrix Concatenation : Concatenate  Mode=Multidimensional array  ConcatenateDimension=2
  Matrix Concatenation1 : Concatenate  Mode=Multidimensional array
  Product{,1..5} (6 alike) : Product  RndMeth=Zero
  Trigonometric Function{,1,6..8,11..12} (7 alike) : Trigonometry  Operator=cos
  Trigonometric Function{2..5,9..10,13} (7 alike) : Trigonometry
  Vector Concatenate : Concatenate  NumInputs=3  Mode=Multidimensional array
  Out1 : Outport
  wires:
  Matrix Concatenation1/1 -> Display/1, Out1/1
  Constant1/1 -> Matrix Concatenation1/2
  Matrix Concatenation/1 -> Matrix Concatenation1/1
  Vector Concatenate/1 -> Matrix Concatenation/2
  A_01/1 -> Matrix Concatenation/1
  Constant5/1 -> Vector Concatenate/3
  Product5/1 -> Vector Concatenate/2
  Product4/1 -> Vector Concatenate/1
  Trigonometric Function13/1 -> Product5/2
  Trigonometric Function12/1 -> Product4/2
  a/1 -> Product5/1, Product4/1
  Trigonometric Function11/1 -> A_01/9
  Trigonometric Function10/1 -> A_01/8
  Constant/1 -> A_01/7
  Gain1/1 -> A_01/6
  Product2/1 -> A_01/5
  Trigonometric Function5/1 -> A_01/4
  Product1/1 -> A_01/3
  Gain/1 -> A_01/2
  Trigonometric Function/1 -> A_01/1
  Product/1 -> Gain/1
  Product3/1 -> Gain1/1
  Trigonometric Function9/1 -> Product3/2
  Trigonometric Function8/1 -> Product3/1
  Trigonometric Function7/1 -> Product2/2
  Trigonometric Function6/1 -> Product2/1
  Trigonometric Function4/1 -> Product1/2
  Trigonometric Function3/1 -> Product1/1
  alpha/1 -> Trigonometric Function11/1, Trigonometric Function10/1, Trigonometric Function9/1, Trigonometric Function7/1, Trigonometric Function4/1, Trigonometric Function1/1
  q/1 -> Trigonometric Function13/1, Trigonometric Function12/1, Trigonometric Function8/1, Trigonometric Function6/1, Trigonometric Function5/1, Trigonometric Function3/1, Trigonometric Function2/1, Trigonometric Function/1
  Trigonometric Function1/1 -> Product/2
  Trigonometric Function2/1 -> Product/1

== system Simulatore_COMAU_UBHAND_IV_manipolazione_interna/Work Space Control/Inverse_kinematic/Inverse_kinematic_comau/R_0index/T_6_index_^-1/Direct_kinematic_index1/Subsystem1 ==
  q : Inport
  alpha : Inport  Port=2
  a : Inport  Port=3
  A_01 : aerolibutil/Create 3x3 Matrix
  Constant : Constant  Value=0
  Constant1 : Constant  Value=[0 0 0 1]  VectorParams1D=off
  Constant5 : Constant  Value=0
  Display : Display
  Gain : Gain  Gain=-1
  Gain1 : Gain  Gain=-1
  Matrix Concatenation : Concatenate  Mode=Multidimensional array  ConcatenateDimension=2
  Matrix Concatenation1 : Concatenate  Mode=Multidimensional array
  Product{,1..5} (6 alike) : Product  RndMeth=Zero
  Trigonometric Function{,1,6..8,11..12} (7 alike) : Trigonometry  Operator=cos
  Trigonometric Function{2..5,9..10,13} (7 alike) : Trigonometry
  Vector Concatenate : Concatenate  NumInputs=3  Mode=Multidimensional array
  Out1 : Outport
  wires:
  Trigonometric Function2/1 -> Product/1
  Trigonometric Function1/1 -> Product/2
  q/1 -> Trigonometric Function/1, Trigonometric Function2/1, Trigonometric Function3/1, Trigonometric Function5/1, Trigonometric Function6/1, Trigonometric Function8/1, Trigonometric Function12/1, Trigonometric Function13/1
  alpha/1 -> Trigonometric Function1/1, Trigonometric Function4/1, Trigonometric Function7/1, Trigonometric Function9/1, Trigonometric Function10/1, Trigonometric Function11/1
  Trigonometric Function3/1 -> Product1/1
  Trigonometric Function4/1 -> Product1/2
  Trigonometric Function6/1 -> Product2/1
  Trigonometric Function7/1 -> Product2/2
  Trigonometric Function8/1 -> Product3/1
  Trigonometric Function9/1 -> Product3/2
  Product3/1 -> Gain1/1
  Product/1 -> Gain/1
  Trigonometric Function/1 -> A_01/1
  Gain/1 -> A_01/2
  Product1/1 -> A_01/3
  Trigonometric Function5/1 -> A_01/4
  Product2/1 -> A_01/5
  Gain1/1 -> A_01/6
  Constant/1 -> A_01/7
  Trigonometric Function10/1 -> A_01/8
  Trigonometric Function11/1 -> A_01/9
  a/1 -> Product5/1, Product4/1
  Trigonometric Function12/1 -> Product4/2
  Trigonometric Function13/1 -> Product5/2
  Product4/1 -> Vector Concatenate/1
  Product5/1 -> Vector Concatenate/2
  Constant5/1 -> Vector Concatenate/3
  A_01/1 -> Matrix Concatenation/1
  Vector Concatenate/1 -> Matrix Concatenation/2
  Matrix Concatenation/1 -> Matrix Concatenation1/1
  Constant1/1 -> Matrix Concatenation1/2
  Matrix Concatenation1/1 -> Out1/1, Display/1

== system Simulatore_COMAU_UBHAND_IV_manipolazione_interna/Work Space Control/Inverse_kinematic/Inverse_kinematic_comau/R_0index/T_6_index_^-1/Direct_kinematic_index1/Subsystem2 ==
  q : Inport
  alpha : Inport  Port=2
  a : Inport  Port=3
  A_01 : aerolibutil/Create 3x3 Matrix
  Constant : Constant  Value=0
  Constant1 : Constant  Value=[0 0 0 1]  VectorParams1D=off
  Constant5 : Constant  Value=0
  Display : Display
  Gain : Gain  Gain=-1
  Gain1 : Gain  Gain=-1
  Matrix Concatenation : Concatenate  Mode=Multidimensional array  ConcatenateDimension=2
  Matrix Concatenation1 : Concatenate  Mode=Multidimensional array
  Product{,1..5} (6 alike) : Product  RndMeth=Zero
  Trigonometric Function{,1,6..8,11..12} (7 alike) : Trigonometry  Operator=cos
  Trigonometric Function{2..5,9..10,13} (7 alike) : Trigonometry
  Vector Concatenate : Concatenate  NumInputs=3  Mode=Multidimensional array
  Out1 : Outport
  wires:
  Matrix Concatenation1/1 -> Display/1, Out1/1
  Constant1/1 -> Matrix Concatenation1/2
  Matrix Concatenation/1 -> Matrix Concatenation1/1
  Vector Concatenate/1 -> Matrix Concatenation/2
  A_01/1 -> Matrix Concatenation/1
  Constant5/1 -> Vector Concatenate/3
  Product5/1 -> Vector Concatenate/2
  Product4/1 -> Vector Concatenate/1
  Trigonometric Function13/1 -> Product5/2
  Trigonometric Function12/1 -> Product4/2
  a/1 -> Product5/1, Product4/1
  Trigonometric Function11/1 -> A_01/9
  Trigonometric Function10/1 -> A_01/8
  Constant/1 -> A_01/7
  Gain1/1 -> A_01/6
  Product2/1 -> A_01/5
  Trigonometric Function5/1 -> A_01/4
  Product1/1 -> A_01/3
  Gain/1 -> A_01/2
  Trigonometric Function/1 -> A_01/1
  Product/1 -> Gain/1
  Product3/1 -> Gain1/1
  Trigonometric Function9/1 -> Product3/2
  Trigonometric Function8/1 -> Product3/1
  Trigonometric Function7/1 -> Product2/2
  Trigonometric Function6/1 -> Product2/1
  Trigonometric Function4/1 -> Product1/2
  Trigonometric Function3/1 -> Product1/1
  alpha/1 -> Trigonometric Function11/1, Trigonometric Function10/1, Trigonometric Function9/1, Trigonometric Function7/1, Trigonometric Function4/1, Trigonometric Function1/1
  q/1 -> Trigonometric Function13/1, Trigonometric Function12/1, Trigonometric Function8/1, Trigonometric Function6/1, Trigonometric Function5/1, Trigonometric Function3/1, Trigonometric Function2/1, Trigonometric Function/1
  Trigonometric Function1/1 -> Product/2
  Trigonometric Function2/1 -> Product/1
